simulink model "TosAeb"  (R2021a)
settings: Solver=FixedStepDiscrete  StartTime=0.0  StopTime=inf  FixedStep=0.01  RelTol=1e-3
undefined symbols (not defined in the file or in its folder): ACCModSts, AccSts1, AEB_ObjectMovingOrientation, AEB_ObjectMovingState, CN_BTCActive, CN_CurrentGearR, CN_CurrentGearR_DHT, CN_PTCActive, CN_VDCActive, CN_VSC_EspOff, CN_VSC_FCW_AEB_Error, CN_VSC_FCW_AEB_NoDispay, CN_VSC_FCW_AEB_PerfmDgdtn, CN_VSC_FCW_AEB_TempUnAvlbl, CN_VSC_VehStandstill, CNaccelAvoidMax, CNaccelAvoidMin, CNbrakePedalPress, CNcomfortStrLeftAccelMax, CNcomfortStrLeftAccelMin, CNcomfortStrRightAccelMax, CNcomfortStrRightAccelMin, CNDrvDoorOpen, CNDrvModeEco, CNDrvModeNorm, CNDrvModeOther, CNDrvModeSport, CNDrvSBRreminder, CNNull, CNobjInvalidID, CNPassDoorOpen, CNPassSBRreminder, CNPEBSAccelNegInf, CNPEBSAccelNull, CNPEBSTimeInf, CNPEBSTimeNull, CNSTOPMAX, CNSTOPNULL, CNsubjWidth, CNSwtOFF, CNSwtON, CNTTCMAX, CNTTCNULL, CNVehConfigAEBCycNone, CNVehConfigAEBNone, CNVehConfigAEBPedNone, CNVehConfigFCWNone, CNVehConfigJANone, CollisionSeverity, FALSE, FCWSnvty, JACycObjCalcInfoDefaultParams, JAPedObjCalcInfoDefaultParams, JAVehObjCalcInfoDefaultParams, latCycObjCalcInfoDefaultParams, latPedObjCalcInfoDefaultParams, longObjCalcInfoDefaultParams, ModSts, movingStatus, ObjectType ... (+1112)
libraries: simulink x3031, AEBSFunctionLib x50, Ta_Library x33, AEBSStateMachineLib x23, Mylibrary x12, simulink_extras x7, ObjSelect_lib x3, dspstat3 x2
(part 65 of 102)

== system TosAeb/FcwAebJa_Step_sys/ALG_AEBSystem/ALG_AEBController/AEB_LongTgtInfoCalc/LongObjCalcInfoCalc/AEBS_LongCollisionAssesApp/collisionSeverityHighCalc/collisionSeverityHighCalc1/BrkSysAtribution/FCWSnvtyLate ==
  ObjectMovingState : Inport
  subjVel : Inport  Port=2
  subjLongAccel : Inport  Port=3
  Action Port : ActionPort  ActionPortLabel=case [ HMISettingLate ]:
  Bus Creator : BusCreator  Inputs=3
  Bus Creator1 : BusCreator  Inputs=3
  Bus Creator2 : BusCreator  Inputs=2
  Compare To Constant : simulink/Logic and Bit Operations/Compare To Constant  relop===  const=AEB_ObjectMovingState.Still  OutDataTypeStr=boolean
  Compare To Constant1 : simulink/Logic and Bit Operations/Compare To Constant  relop===  const=AEB_ObjectMovingState.Still  OutDataTypeStr=boolean
  Compare To Constant2 : simulink/Logic and Bit Operations/Compare To Constant  relop===  const=AEB_ObjectMovingState.Still  OutDataTypeStr=boolean
  Constant : Constant  Commented=on  Value=P_AEB_LongCA_SeverityHighDlyTimeConfig
  DelayTimeHigh : Constant  Commented=on  Value=P_AEB_LongCA_SeverityHighBrkDlyTimeHighHmiLate  OutDataTypeStr=single
  DelayTimeLow : Constant  Commented=on  Value=P_AEB_LongCA_SeverityHighBrkDlyTimeLowHmiLate  OutDataTypeStr=single
  SubjAccelThres : simulink/Logic and Bit Operations/Compare To Constant  relop=<  const=P_AEB_LongCA_SeverityHighSubjAccelThes4BrkDlyTimeHmiLate  OutDataTypeStr=boolean
  Switch{,2..4} (4 alike) : Switch  Criteria=u2 ~= 0
  Switch1 : Switch  Commented=on  Criteria=u2 ~= 0
  VelMaxReudceMO : Constant  Value=P_AEB_LongCA_SeverityHighVelMaxReduceMOHmiLate
  VelMaxReudceSO : Constant  Value=P_AEB_LongCA_SeverityHighVelMaxReduceSOHmiLate
  accelGradientMOLookUp : Lookup_n-D  NumberOfTableDimensions=1  BreakpointsForDimension1=P_AEB_LongCA_SeverityHighAccelGredientMOXHmiLate  BeginIndexSearchUsingPreviousIndexResult=on  Table=P_AEB_LongCA_SeverityHighAccelGredientMOYHmiLate  ExtrapMethod=Clip  InternalRulePriority=Speed  RndMeth=Simplest  InputSameDT=on
  accelGradientSOLookUp : Lookup_n-D  NumberOfTableDimensions=1  BreakpointsForDimension1=P_AEB_LongCA_SeverityHighAccelGredientSOXHmiLate  BeginIndexSearchUsingPreviousIndexResult=on  Table=P_AEB_LongCA_SeverityHighAccelGredientSOYHmiLate  ExtrapMethod=Clip  InternalRulePriority=Speed  RndMeth=Simplest  InputSameDT=on
  accelMaxReudceMOLookUp : Lookup_n-D  NumberOfTableDimensions=1  BreakpointsForDimension1=P_AEB_LongCA_SeverityHighAccelMaxDecMOXHmiLate  BeginIndexSearchUsingPreviousIndexResult=on  Table=P_AEB_LongCA_SeverityHighAccelMaxDecMOYHmiLate  ExtrapMethod=Clip  InternalRulePriority=Speed  RndMeth=Simplest  InputSameDT=on
  accelMaxReudceSOLookUp : Lookup_n-D  NumberOfTableDimensions=1  BreakpointsForDimension1=P_AEB_LongCA_SeverityHighAccelMaxDecSOXHmiLate  BeginIndexSearchUsingPreviousIndexResult=on  Table=P_AEB_LongCA_SeverityHighAccelMaxDecSOYHmiLate  ExtrapMethod=Clip  InternalRulePriority=Speed  RndMeth=Simplest  InputSameDT=on
  tBrakeLatencyHighMOLookUp : Lookup_n-D  NumberOfTableDimensions=1  BreakpointsForDimension1=P_AEB_LongCA_SeverityHighDlyTimeMOXHmiLate  BeginIndexSearchUsingPreviousIndexResult=on  Table=P_AEB_LongCA_SeverityHighDlyTimeMOYHmiLate  ExtrapMethod=Clip  InternalRulePriority=Speed  RndMeth=Simplest  InputSameDT=on
  tBrakeLatencyHighSOLookUp : Lookup_n-D  NumberOfTableDimensions=1  BreakpointsForDimension1=P_AEB_LongCA_SeverityHighDlyTimeSOXHmiLate  BeginIndexSearchUsingPreviousIndexResult=on  Table=P_AEB_LongCA_SeverityHighDlyTimeSOYHmiLate  ExtrapMethod=Clip  InternalRulePriority=Speed  RndMeth=Simplest  InputSameDT=on
  tBrakeLatencyLowMOLookUp : Lookup_n-D  NumberOfTableDimensions=1  BreakpointsForDimension1=P_AEB_LongCA_SeverityHighDlyTimeLowMOXHmiLate  BeginIndexSearchUsingPreviousIndexResult=on  Table=P_AEB_LongCA_SeverityHighDlyTimeLowMOYHmiLate  ExtrapMethod=Clip  InternalRulePriority=Speed  RndMeth=Simplest  InputSameDT=on
  tBrakeLatencyLowSOLookUp : Lookup_n-D  NumberOfTableDimensions=1  BreakpointsForDimension1=P_AEB_LongCA_SeverityHighDlyTimeLowSOXHmiLate  BeginIndexSearchUsingPreviousIndexResult=on  Table=P_AEB_LongCA_SeverityHighDlyTimeLowSOYHmiLate  ExtrapMethod=Clip  InternalRulePriority=Speed  RndMeth=Simplest  InputSameDT=on
  brkSys : Outport
  wires:
  Bus Creator2/1 -> brkSys/1
  accelMaxReudceMOLookUp/1 -> Bus Creator1/2  "accelMaxReudce"
  accelGradientMOLookUp/1 -> Bus Creator1/3  "accelGradient"
  VelMaxReudceMO/1 -> Bus Creator1/1  "velMaxReudce"
  Compare To Constant/1 -> Switch/2
  VelMaxReudceSO/1 -> Bus Creator/1  "velMaxReudce"
  Bus Creator/1 -> Switch/1
  Switch/1 -> Bus Creator2/1  "brkSysAtribution"
  Switch3/1 -> Bus Creator2/2  "tBrakeLatency"
  subjLongAccel/1 -> SubjAccelThres/1
  accelGradientSOLookUp/1 -> Bus Creator/3  "accelGradient"
  Bus Creator1/1 -> Switch/3
  ObjectMovingState/1 -> [ObjectMovingState], Compare To Constant/1
  accelMaxReudceSOLookUp/1 -> Bus Creator/2  "accelMaxReudce"
  [ObjectMovingState] -> Compare To Constant1/1
  subjVel/1 -> accelMaxReudceSOLookUp/1, accelGradientSOLookUp/1, accelMaxReudceMOLookUp/1, accelGradientMOLookUp/1, tBrakeLatencyHighSOLookUp/1, tBrakeLatencyHighMOLookUp/1, tBrakeLatencyLowMOLookUp/1, tBrakeLatencyLowSOLookUp/1
  tBrakeLatencyHighMOLookUp/1 -> Switch2/3
  Compare To Constant1/1 -> Switch2/2
  tBrakeLatencyHighSOLookUp/1 -> Switch2/1
  Switch2/1 -> Switch3/3  "tBrakeLatency"
  SubjAccelThres/1 -> Switch3/2
  tBrakeLatencyLowSOLookUp/1 -> Switch4/1
  Compare To Constant2/1 -> Switch4/2
  tBrakeLatencyLowMOLookUp/1 -> Switch4/3
  [ObjectMovingState] -> Compare To Constant2/1
  Switch4/1 -> Switch3/1  "tBrakeLatency"

== system TosAeb/FcwAebJa_Step_sys/ALG_AEBSystem/ALG_AEBController/AEB_LongTgtInfoCalc/LongObjCalcInfoCalc/AEBS_LongCollisionAssesApp/collisionSeverityHighCalc/collisionSeverityHighCalc1/BrkSysAtribution/FCWSnvtyMid ==
  ObjectMovingState : Inport
  subjVel : Inport  Port=2
  subjLongAccel : Inport  Port=3
  Action Port : ActionPort  ActionPortLabel=default:
  Bus Creator : BusCreator  Inputs=3
  Bus Creator1 : BusCreator  Inputs=3
  Bus Creator2 : BusCreator  Inputs=2
  Compare To Constant : simulink/Logic and Bit Operations/Compare To Constant  relop===  const=AEB_ObjectMovingState.Still  OutDataTypeStr=boolean
  Compare To Constant1 : simulink/Logic and Bit Operations/Compare To Constant  relop===  const=AEB_ObjectMovingState.Still  OutDataTypeStr=boolean
  Compare To Constant2 : simulink/Logic and Bit Operations/Compare To Constant  relop===  const=AEB_ObjectMovingState.Still  OutDataTypeStr=boolean
  Constant : Constant  Commented=on  Value=P_AEB_LongCA_SeverityHighDlyTimeConfig
  DelayTimeHigh : Constant  Commented=on  Value=P_AEB_LongCA_SeverityHighBrkDlyTimeHighHmiMid  OutDataTypeStr=single
  DelayTimeLow : Constant  Commented=on  Value=P_AEB_LongCA_SeverityHighBrkDlyTimeLowHmiMid  OutDataTypeStr=single
  SubjAccelThres : simulink/Logic and Bit Operations/Compare To Constant  relop=<  const=P_AEB_LongCA_SeverityHighSubjAccelThes4BrkDlyTimeHmiMid  OutDataTypeStr=boolean
  Switch{,2..4} (4 alike) : Switch  Criteria=u2 ~= 0
  Switch1 : Switch  Commented=on  Criteria=u2 ~= 0
  VelMaxReudceMO : Constant  Value=P_AEB_LongCA_SeverityHighVelMaxReduceMOHmiMid
  VelMaxReudceSO : Constant  Value=P_AEB_LongCA_SeverityHighVelMaxReduceSOHmiMid
  accelGradientMOLookUp : Lookup_n-D  NumberOfTableDimensions=1  BreakpointsForDimension1=P_AEB_LongCA_SeverityHighAccelGredientMOXHmiMid  BeginIndexSearchUsingPreviousIndexResult=on  Table=P_AEB_LongCA_SeverityHighAccelGredientMOYHmiMid  ExtrapMethod=Clip  InternalRulePriority=Speed  RndMeth=Simplest  InputSameDT=on
  accelGradientSOLookUp : Lookup_n-D  NumberOfTableDimensions=1  BreakpointsForDimension1=P_AEB_LongCA_SeverityHighAccelGredientSOXHmiMid  BeginIndexSearchUsingPreviousIndexResult=on  Table=P_AEB_LongCA_SeverityHighAccelGredientSOYHmiMid  ExtrapMethod=Clip  InternalRulePriority=Speed  RndMeth=Simplest  InputSameDT=on
  accelMaxReudceMOLookUp : Lookup_n-D  NumberOfTableDimensions=1  BreakpointsForDimension1=P_AEB_LongCA_SeverityHighAccelMaxDecMOXHmiMid  BeginIndexSearchUsingPreviousIndexResult=on  Table=P_AEB_LongCA_SeverityHighAccelMaxDecMOYHmiMid  ExtrapMethod=Clip  InternalRulePriority=Speed  RndMeth=Simplest  InputSameDT=on
  accelMaxReudceSOLookUp : Lookup_n-D  NumberOfTableDimensions=1  BreakpointsForDimension1=P_AEB_LongCA_SeverityHighAccelMaxDecSOXHmiMid  BeginIndexSearchUsingPreviousIndexResult=on  Table=P_AEB_LongCA_SeverityHighAccelMaxDecSOYHmiMid  ExtrapMethod=Clip  InternalRulePriority=Speed  RndMeth=Simplest  InputSameDT=on
  tBrakeLatencyLowMOLookUp : Lookup_n-D  NumberOfTableDimensions=1  BreakpointsForDimension1=P_AEB_LongCA_SeverityHighDlyTimeLowMOXHmiMid  BeginIndexSearchUsingPreviousIndexResult=on  Table=P_AEB_LongCA_SeverityHighDlyTimeLowMOYHmiMid  ExtrapMethod=Clip  InternalRulePriority=Speed  RndMeth=Simplest  InputSameDT=on
  tBrakeLatencyLowSOLookUp : Lookup_n-D  NumberOfTableDimensions=1  BreakpointsForDimension1=P_AEB_LongCA_SeverityHighDlyTimeLowSOXHmiMid  BeginIndexSearchUsingPreviousIndexResult=on  Table=P_AEB_LongCA_SeverityHighDlyTimeLowSOYHmiMid  ExtrapMethod=Clip  InternalRulePriority=Speed  RndMeth=Simplest  InputSameDT=on
  tBrakeLatencyMOLookUp : Lookup_n-D  NumberOfTableDimensions=1  BreakpointsForDimension1=P_AEB_LongCA_SeverityHighDlyTimeMOXHmiMid  BeginIndexSearchUsingPreviousIndexResult=on  Table=P_AEB_LongCA_SeverityHighDlyTimeMOYHmiMid  ExtrapMethod=Clip  InternalRulePriority=Speed  RndMeth=Simplest  InputSameDT=on
  tBrakeLatencySOLookUp : Lookup_n-D  NumberOfTableDimensions=1  BreakpointsForDimension1=P_AEB_LongCA_SeverityHighDlyTimeSOXHmiMid  BeginIndexSearchUsingPreviousIndexResult=on  Table=P_AEB_LongCA_SeverityHighDlyTimeSOYHmiMid  ExtrapMethod=Clip  InternalRulePriority=Speed  RndMeth=Simplest  InputSameDT=on
  brkSys : Outport
  wires:
  subjVel/1 -> tBrakeLatencyMOLookUp/1, tBrakeLatencySOLookUp/1, tBrakeLatencyLowMOLookUp/1, tBrakeLatencyLowSOLookUp/1, accelGradientMOLookUp/1, accelMaxReudceMOLookUp/1, accelGradientSOLookUp/1, accelMaxReudceSOLookUp/1
  accelMaxReudceSOLookUp/1 -> Bus Creator/2  "accelMaxReudce"
  ObjectMovingState/1 -> [ObjectMovingState], Compare To Constant/1
  Bus Creator1/1 -> Switch/3
  accelGradientSOLookUp/1 -> Bus Creator/3  "accelGradient"
  subjLongAccel/1 -> SubjAccelThres/1
  Switch3/1 -> Bus Creator2/2  "tBrakeLatency"
  Switch/1 -> Bus Creator2/1  "brkSysAtribution"
  Bus Creator/1 -> Switch/1
  VelMaxReudceSO/1 -> Bus Creator/1  "velMaxReudce"
  Compare To Constant/1 -> Switch/2
  VelMaxReudceMO/1 -> Bus Creator1/1  "velMaxReudce"
  accelGradientMOLookUp/1 -> Bus Creator1/3  "accelGradient"
  accelMaxReudceMOLookUp/1 -> Bus Creator1/2  "accelMaxReudce"
  Bus Creator2/1 -> brkSys/1
  Compare To Constant1/1 -> Switch2/2
  tBrakeLatencySOLookUp/1 -> Switch2/1
  tBrakeLatencyMOLookUp/1 -> Switch2/3
  [ObjectMovingState] -> Compare To Constant1/1
  Switch2/1 -> Switch3/3  "tBrakeLatency"
  SubjAccelThres/1 -> Switch3/2
  Switch4/1 -> Switch3/1  "tBrakeLatency"
  [ObjectMovingState] -> Compare To Constant2/1
  tBrakeLatencyLowMOLookUp/1 -> Switch4/3
  tBrakeLatencyLowSOLookUp/1 -> Switch4/1
  Compare To Constant2/1 -> Switch4/2

== system TosAeb/FcwAebJa_Step_sys/ALG_AEBSystem/ALG_AEBController/AEB_LongTgtInfoCalc/LongObjCalcInfoCalc/AEBS_LongCollisionAssesApp/collisionSeverityHighCalc/collisionSeverityHighCalc1/CollisionAssessHighCalcVersion3/CollisionAssessHighCalc1 ==
  accelMaxDec : Inport
  accelGradient : Inport  Port=2
  velMaxReudce : Inport  Port=3
  tBrakeLatency : Inport  Port=4
  vxAbsObj : Inport  Port=5
  axAbsObj : Inport  Port=6
  subjVel : Inport  Port=7
  subjLongAccel : Inport  Port=8
  dxFrontBumper : Inport  Port=9
  dxSecure : Inport  Port=10
  Action Port : ActionPort  ActionPortLabel=else
  Bus Creator : BusCreator  Inputs=8
  Bus Selector : BusSelector  OutputSignals=subjLongAccelFilt,axAbsObj,tBrakeLatency,velMaxReudce,subjVel,vxAbsObj
  Bus Selector2 : BusSelector  OutputSignals=accelMaxDec,subjLongAccelFilt,accelGradient,axAbsObj
  Bus Selector3 : BusSelector  OutputSignals=accelMaxDec,axAbsObj,accelMaxDec
  CalcBrakePhase1 : Subsystem
  CalcBrakePhase2 : Subsystem
  CalcBrakePhase3 : Subsystem
  collisionSeverityHighFlgJudge : Subsystem
  dxWithoutSecureCalc : Subsystem
  subjLongAccelFiltCalc : Subsystem
  collisionSeverityHighFlg : Outport
  wires:
  Bus Selector3/3 -> CalcBrakePhase3/3(accelMaxDec)  "<accelMaxDec>"
  Bus Selector2/1 -> CalcBrakePhase2/1(accelMaxDec)  "<accelMaxDec>"
  tBrakeLatency/1 -> Bus Creator/4  "tBrakeLatency"
  velMaxReudce/1 -> Bus Creator/3  "velMaxReudce"
  accelGradient/1 -> Bus Creator/2  "accelGradient"
  accelMaxDec/1 -> Bus Creator/1  "accelMaxDec"
  subjLongAccelFiltCalc/1(subjLongAccelFilt) -> Bus Creator/8  "subjLongAccelFilt"
  subjVel/1 -> Bus Creator/7  "subjVel"
  axAbsObj/1 -> Bus Creator/6  "axAbsObj"
  vxAbsObj/1 -> Bus Creator/5  "vxAbsObj"
  Bus Selector3/1 -> CalcBrakePhase3/1(a0Ego)  "<accelMaxDec>"
  subjLongAccel/1 -> subjLongAccelFiltCalc/1(subjLongAccel)
  dxWithoutSecureCalc/1(dxWithoutSecure) -> CalcBrakePhase1/7(dx)
  CalcBrakePhase1/4(vtObj) -> CalcBrakePhase2/7(v0Obj)
  CalcBrakePhase1/3(vtEgo) -> CalcBrakePhase2/6(v0Ego)
  [egoCollisionFlgPhase1] -> collisionSeverityHighFlgJudge/1(egoCollisionFlgPhase1)
  [egoCollisionFlgPhase2] -> collisionSeverityHighFlgJudge/2(egoCollisionFlgPhase2)
  CalcBrakePhase3/1(egoCollisionFlg) -> collisionSeverityHighFlgJudge/3(egoCollisionFlgPhase3)
  collisionSeverityHighFlgJudge/1(collisionSeverityHighFlg) -> collisionSeverityHighFlg/1
  CalcBrakePhase1/1(egoCollisionFlg) -> [egoCollisionFlgPhase1]
  CalcBrakePhase2/1(egoCollisionFlg) -> [egoCollisionFlgPhase2]
  CalcBrakePhase2/4(vtObj) -> CalcBrakePhase3/6(v0Obj)
  CalcBrakePhase2/3(vtEgo) -> CalcBrakePhase3/5(v0Ego)
  dxFrontBumper/1 -> dxWithoutSecureCalc/1(dxFrontBumper)
  dxSecure/1 -> dxWithoutSecureCalc/2(dxSecure)
  Bus Creator/1 -> Bus Selector/1, Bus Selector2/1, Bus Selector3/1
  Bus Selector/3 -> CalcBrakePhase1/3(tBrakeLatency)  "<tBrakeLatency>"
  Bus Selector/2 -> CalcBrakePhase1/2(a0Obj)  "<axAbsObj>"
  Bus Selector/4 -> CalcBrakePhase1/4(velMaxReudce)  "<velMaxReudce>"
  Bus Selector/1 -> CalcBrakePhase1/1(a0Ego)  "<subjLongAccelFilt>"
  Bus Selector/5 -> CalcBrakePhase1/5(v0Ego)  "<subjVel>"
  Bus Selector/6 -> CalcBrakePhase1/6(v0Obj)  "<vxAbsObj>"
  Bus Selector2/2 -> CalcBrakePhase2/2(a0Ego)  "<subjLongAccelFilt>"
  Bus Selector2/3 -> CalcBrakePhase2/3(accelGradient)  "<accelGradient>"
  Bus Selector2/4 -> CalcBrakePhase2/4(a0Obj)  "<axAbsObj>"
  Bus Selector3/2 -> CalcBrakePhase3/2(a0Obj)  "<axAbsObj>"
  CalcBrakePhase1/2(restDeltaVel) -> CalcBrakePhase2/5(velMaxReudce)
  CalcBrakePhase2/2(restDeltaVelocity) -> CalcBrakePhase3/4(velMaxReudce)
  CalcBrakePhase1/5(dxt) -> CalcBrakePhase2/8(dx)
  CalcBrakePhase2/5(dxt) -> CalcBrakePhase3/7(dx0)

== system TosAeb/FcwAebJa_Step_sys/ALG_AEBSystem/ALG_AEBController/AEB_LongTgtInfoCalc/LongObjCalcInfoCalc/AEBS_LongCollisionAssesApp/collisionSeverityHighCalc/collisionSeverityHighCalc1/CollisionAssessHighCalcVersion3/CollisionAssessHighCalc2 ==
  accelMaxDec : Inport
  accelGradient : Inport  Port=2
  velMaxReudce : Inport  Port=3
  tBrakeLatency : Inport  Port=4
  vxAbsObj : Inport  Port=5
  axAbsObj : Inport  Port=6
  subjVel : Inport  Port=7
  subjLongAccel : Inport  Port=8
  dxFrontBumper : Inport  Port=9
  dxSecure : Inport  Port=10
  Action Port : ActionPort
  Bus Creator : BusCreator  Inputs=8
  Bus Selector : BusSelector  OutputSignals=subjLongAccelFilt,axAbsObj,tBrakeLatency,velMaxReudce,subjVel,vxAbsObj
  Bus Selector2 : BusSelector  OutputSignals=accelMaxDec,subjLongAccelFilt,accelGradient,axAbsObj
  Bus Selector3 : BusSelector  OutputSignals=accelMaxDec,axAbsObj,accelMaxDec
  CalcBrakePhase1 : Subsystem
  CalcBrakePhase2 : Subsystem
  CalcBrakePhase3 : Subsystem
  Constant : Constant  Value=0
  Constant2 : Constant  Value=0
  collisionSeverityHighFlgJudge : Subsystem
  dxWithoutSecureCalc : Subsystem
  subjLongAccelFiltCalc : Subsystem
  collisionSeverityHighFlg : Outport
  wires:
  Bus Selector3/3 -> CalcBrakePhase3/3(accelMaxDec)  "<accelMaxDec>"
  Bus Selector2/1 -> CalcBrakePhase2/1(accelMaxDec)  "<accelMaxDec>"
  tBrakeLatency/1 -> Bus Creator/4  "tBrakeLatency"
  velMaxReudce/1 -> Bus Creator/3  "velMaxReudce"
  accelGradient/1 -> Bus Creator/2  "accelGradient"
  accelMaxDec/1 -> Bus Creator/1  "accelMaxDec"
  subjLongAccelFiltCalc/1(subjLongAccelFilt) -> Bus Creator/8  "subjLongAccelFilt"
  subjVel/1 -> Bus Creator/7  "subjVel"
  axAbsObj/1 -> Bus Creator/6  "axAbsObj"
  vxAbsObj/1 -> Bus Creator/5  "vxAbsObj"
  Bus Selector3/1 -> CalcBrakePhase3/1(a0Ego)  "<accelMaxDec>"
  subjLongAccel/1 -> subjLongAccelFiltCalc/1(subjLongAccel)
  dxWithoutSecureCalc/1(dxWithoutSecure) -> CalcBrakePhase1/9(dx), CalcBrakePhase3/9(dx), CalcBrakePhase2/10(dx)
  CalcBrakePhase1/6(stObj) -> CalcBrakePhase2/9(s0Obj)
  CalcBrakePhase1/5(vtObj) -> CalcBrakePhase2/8(v0Obj)
  CalcBrakePhase1/3(vtEgo) -> CalcBrakePhase2/6(v0Ego)
  CalcBrakePhase1/4(stEgo) -> CalcBrakePhase2/7(s0Ego)
  [egoCollisionFlgPhase1] -> collisionSeverityHighFlgJudge/1(egoCollisionFlgPhase1)
  [egoCollisionFlgPhase2] -> collisionSeverityHighFlgJudge/2(egoCollisionFlgPhase2)
  CalcBrakePhase3/1(egoCollisionFlg) -> collisionSeverityHighFlgJudge/3(egoCollisionFlgPhase3)
  collisionSeverityHighFlgJudge/1(collisionSeverityHighFlg) -> collisionSeverityHighFlg/1
  CalcBrakePhase1/1(egoCollisionFlg) -> [egoCollisionFlgPhase1]
  CalcBrakePhase2/1(egoCollisionFlg) -> [egoCollisionFlgPhase2]
  CalcBrakePhase2/6(stObj) -> CalcBrakePhase3/8(s0Obj)
  CalcBrakePhase2/5(vtObj) -> CalcBrakePhase3/7(v0Obj)
  CalcBrakePhase2/4(stEgo) -> CalcBrakePhase3/6(s0Ego)
  CalcBrakePhase2/3(vtEgo) -> CalcBrakePhase3/5(v0Ego)
  Constant/1 -> CalcBrakePhase1/8(s0Obj)
  Constant2/1 -> CalcBrakePhase1/6(s0Ego)
  dxFrontBumper/1 -> dxWithoutSecureCalc/1(dxFrontBumper)
  dxSecure/1 -> dxWithoutSecureCalc/2(dxSecure)
  Bus Creator/1 -> Bus Selector/1, Bus Selector2/1, Bus Selector3/1
  Bus Selector/3 -> CalcBrakePhase1/3(tBrakeLatency)  "<tBrakeLatency>"
  Bus Selector/2 -> CalcBrakePhase1/2(a0Obj)  "<axAbsObj>"
  Bus Selector/4 -> CalcBrakePhase1/4(velMaxReudce)  "<velMaxReudce>"
  Bus Selector/1 -> CalcBrakePhase1/1(a0Ego)  "<subjLongAccelFilt>"
  Bus Selector/5 -> CalcBrakePhase1/5(v0Ego)  "<subjVel>"
  Bus Selector/6 -> CalcBrakePhase1/7(v0Obj)  "<vxAbsObj>"
  Bus Selector2/2 -> CalcBrakePhase2/2(a0Ego)  "<subjLongAccelFilt>"
  Bus Selector2/3 -> CalcBrakePhase2/3(accelGradient)  "<accelGradient>"
  Bus Selector2/4 -> CalcBrakePhase2/4(a0Obj)  "<axAbsObj>"
  Bus Selector3/2 -> CalcBrakePhase3/2(a0Obj)  "<axAbsObj>"
  CalcBrakePhase1/2(restDeltaVel) -> CalcBrakePhase2/5(velMaxReudce)
  CalcBrakePhase2/2(restDeltaVelocity) -> CalcBrakePhase3/4(velMaxReudce)

== system TosAeb/FcwAebJa_Step_sys/ALG_AEBSystem/ALG_AEBController/AEB_LongTgtInfoCalc/LongObjCalcInfoCalc/AEBS_LongCollisionAssesApp/collisionSeverityHighCalc/collisionSeverityHighCalc1/CollisionAssessHighCalcVersion3/CollisionAssessHighCalcInvalid ==
  accelGradient : Inport
  accelMaxDec : Inport  Port=2
  Compare To Constant : simulink/Logic and Bit Operations/Compare To Constant  relop=>=  const=CNNull  OutDataTypeStr=boolean
  Compare To Constant1 : simulink/Logic and Bit Operations/Compare To Constant  relop=>=  const=CNNull  OutDataTypeStr=boolean
  Logical Operator : Logic  Operator=OR  AllPortsSameDT=off  OutDataTypeStr=boolean
  Invalid : Outport
  wires:
  Logical Operator/1 -> Invalid/1
  accelGradient/1 -> Compare To Constant/1
  accelMaxDec/1 -> Compare To Constant1/1
  Compare To Constant/1 -> Logical Operator/1
  Compare To Constant1/1 -> Logical Operator/2

== system TosAeb/FcwAebJa_Step_sys/ALG_AEBSystem/ALG_AEBController/AEB_LongTgtInfoCalc/LongObjCalcInfoCalc/AEBS_LongCollisionAssesApp/collisionSeverityHighCalc/collisionSeverityHighCalc1/CollisionAssessHighCalcVersion3/If Action Subsystem ==
  Action Port : ActionPort  ActionPortLabel=if(u1)
  Constant : Constant  Value=false
  collisionSeverityHighFlg : Outport
  wires:
  Constant/1 -> collisionSeverityHighFlg/1

== system TosAeb/FcwAebJa_Step_sys/ALG_AEBSystem/ALG_AEBController/AEB_LongTgtInfoCalc/LongObjCalcInfoCalc/AEBS_LongCollisionAssesApp/collisionSeverityHighCalc/collisionSeverityHighCalc2/collisionSeverityHighPhase1/CollisionSeverityHighPhase1Judge ==
  note: if distEgophase1 > (distObjphase1 + dxRelTargetObjCalc - dxSecure)
      | 	collisionDangerLevel3Fullfilled = true;
      | else
      | 	collisionDangerLevel3Fullfilled = false;
  distEgophase1 : Inport
  distObjphase1 : Inport  Port=2
  dxFrontBumper : Inport  Port=3
  dxSecure : Inport  Port=4
  Add : Sum  Inputs=++-
  Relational Operator : RelationalOperator  Operator=>  OutDataTypeStr=boolean  RndMeth=Simplest
  collisionSeverityHighFlgPhase1 : Outport
  wires:
  dxFrontBumper/1 -> Add/2  "dxRelTargetObjCalc"
  distObjphase1/1 -> Add/1  "distObjphase1"
  dxSecure/1 -> Add/3  "dxSecure"
  Add/1 -> Relational Operator/2
  distEgophase1/1 -> Relational Operator/1  "distEgophase1"
  Relational Operator/1 -> collisionSeverityHighFlgPhase1/1  "collisionSeverityHighFlgPhase1"

== system TosAeb/FcwAebJa_Step_sys/ALG_AEBSystem/ALG_AEBController/AEB_LongTgtInfoCalc/LongObjCalcInfoCalc/AEBS_LongCollisionAssesApp/collisionSeverityHighCalc/collisionSeverityHighCalc2/collisionSeverityHighPhase1/Phase1DistCalc ==
  tWorkPhase1 : Inport
  tObjStopPhase1 : Inport  Port=2
  subjVel : Inport  Port=3
  subjLongAccel : Inport  Port=4
  vxAbsObj : Inport  Port=5
  axAbsObj : Inport  Port=6
  Add : Sum
  Add1 : Sum
  DocBlock : simulink/Model-Wide Utilities/DocBlock  DocumentType=Text
      content=|
      | Type your documentation here
  Gain : Gain  Gain=0.5
  Gain1 : Gain  Gain=0.5
  Math Function2 : Math  Operator=square  SignedPower=on  SaturateOnIntegerOverflow=on
  Math Function3 : Math  Operator=square  SignedPower=on  SaturateOnIntegerOverflow=on
  MinMax : MinMax  Inputs=2
  Product{,1..3} (4 alike) : Product
  distEgoPhase1 : Outport
  distTargetPhase1 : Outport  Port=2
  wires:
  subjLongAccel/1 -> Product/2  "subjAccel"
  tWorkPhase1/1 -> Math Function2/1, Product1/2, MinMax/1  "tWorkPhase1"
  Product/1 -> Gain/1
  subjVel/1 -> Product1/1  "subjVel"
  Gain/1 -> Add/2
  Product1/1 -> Add/1
  tObjStopPhase1/1 -> MinMax/2  "tObjStop"
  Add/1 -> distEgoPhase1/1  "distEgophase1"
  Product3/1 -> Add1/1
  Gain1/1 -> Add1/2
  vxAbsObj/1 -> Product3/1  "vxAbsTargetObjCalc"
  Product2/1 -> Gain1/1
  MinMax/1 -> Math Function3/1, Product3/2  "tWorkPhase1Target"
  axAbsObj/1 -> Product2/2  "axAbsTargetObjCalc"
  Add1/1 -> distTargetPhase1/1  "distTargetphase1"
  Math Function2/1 -> Product/1
  Math Function3/1 -> Product2/1

== system TosAeb/FcwAebJa_Step_sys/ALG_AEBSystem/ALG_AEBController/AEB_LongTgtInfoCalc/LongObjCalcInfoCalc/AEBS_LongCollisionAssesApp/collisionSeverityHighCalc/collisionSeverityHighCalc2/collisionSeverityHighPhase1/Phase1TimeCalc ==
  velMaxReudce : Inport
  subjLongAccel : Inport  Port=2
  subjVel : Inport  Port=3
  vxAbsObj : Inport  Port=4
  axAbsObj : Inport  Port=5
  BrakeLatencyTimeCalc : Subsystem
  Bus Creator : BusCreator  Inputs=5
  Bus Selector1 : BusSelector  OutputSignals=subjAccel,velMaxReudce,subjVel
  Bus Selector3 : BusSelector  OutputSignals=subjAccel,subjVel
  Bus Selector4 : BusSelector  OutputSignals=subjAccel
  Bus Selector6 : BusSelector  OutputSignals=axAbsTargetObjCalc,vxAbsTargetObjCalc
  TimeWorkPhase1Calc : Subsystem
  tEgoStopWorkPhase1 : Subsystem
  tMaxReducePhase1Calc : Subsystem
  tObjStopCalc : Subsystem  TreatAsAtomicUnit=on
  tWorkPhase1 : Outport
  tObjStopPhase1 : Outport  Port=2
  wires:
  tEgoStopWorkPhase1/1(tEgoStop) -> TimeWorkPhase1Calc/2(tEgoStop)  "tEgoStop"
  Bus Selector3/2 -> tEgoStopWorkPhase1/2(subjVel)  "<subjVel>"
  Bus Selector3/1 -> tEgoStopWorkPhase1/1(subjLongAccel)  "<subjAccel>"
  tMaxReducePhase1Calc/1(tMaxReduce) -> TimeWorkPhase1Calc/1(tMaxReduce)  "tMaxReduce"
  Bus Selector1/3 -> tMaxReducePhase1Calc/3(subjVel)  "<subjVel>"
  Bus Selector1/2 -> tMaxReducePhase1Calc/2(velMaxReudce)  "<velMaxReudce>"
  Bus Selector1/1 -> tMaxReducePhase1Calc/1(subjLongAccel)  "<subjAccel>"
  tObjStopCalc/1(tStop) -> tObjStopPhase1/1
  subjVel/1 -> Bus Creator/3  "subjVel"
  subjLongAccel/1 -> Bus Creator/2  "subjAccel"
  velMaxReudce/1 -> Bus Creator/1  "velMaxReudce"
  vxAbsObj/1 -> Bus Creator/4  "vxAbsTargetObjCalc"
  axAbsObj/1 -> Bus Creator/5  "axAbsTargetObjCalc"
  Bus Creator/1 -> Bus Selector1/1, Bus Selector3/1, Bus Selector4/1, Bus Selector6/1
  Bus Selector6/2 -> tObjStopCalc/2(velAbs)  "<vxAbsTargetObjCalc>"
  Bus Selector6/1 -> tObjStopCalc/1(accelAbs)  "<axAbsTargetObjCalc>"
  Bus Selector4/1 -> BrakeLatencyTimeCalc/1(subjLongAccel)  "<subjAccel>"
  BrakeLatencyTimeCalc/1(tBrakeLatency) -> TimeWorkPhase1Calc/3(tBrakeLatency)  "tBrakeLatency"
  TimeWorkPhase1Calc/1(tWorkPhase1) -> tWorkPhase1/1

== system TosAeb/FcwAebJa_Step_sys/ALG_AEBSystem/ALG_AEBController/AEB_LongTgtInfoCalc/LongObjCalcInfoCalc/AEBS_LongCollisionAssesApp/collisionSeverityHighCalc/collisionSeverityHighCalc2/collisionSeverityHighPhase2/DistPhase2Calc ==
  accelGradient : Inport
  subVelWorkPhase1 : Inport  Port=2
  subjLongAccelWorkPhase1 : Inport  Port=3
  vxAbsWorkPhase1Target : Inport  Port=4
  axAbsWorkPhase1Target : Inport  Port=5
  tWorkPhase2 : Inport  Port=6
  tObjStopWorkPhase2 : Inport  Port=7
  Add : Sum  Inputs=+++
  Add1 : Sum
  Gain : Gain  Gain=0.5
  Gain1 : Gain  Gain=0.5
  Gain2 : Gain  Gain=1/6
  Math Function2 : Math  Operator=square  SignedPower=on  SaturateOnIntegerOverflow=on
  Math Function3 : Math  Operator=square  SignedPower=on  SaturateOnIntegerOverflow=on
  MinMax : MinMax  Inputs=2
  Product{,1..3} (4 alike) : Product
  Product4 : Product  Inputs=4
  distEgophase2 : Outport
  distTargetphase2 : Outport  Port=2
  wires:
  subjLongAccelWorkPhase1/1 -> Product/2
  tWorkPhase2/1 -> Product4/2, Math Function2/1, MinMax/1, Product1/2, Product4/4, Product4/3
  Product/1 -> Gain/1
  Gain/1 -> Add/3
  Product1/1 -> Add/2
  tObjStopWorkPhase2/1 -> MinMax/2
  Add/1 -> distEgophase2/1
  Product3/1 -> Add1/1
  Gain1/1 -> Add1/2
  vxAbsWorkPhase1Target/1 -> Product3/1
  Product2/1 -> Gain1/1
  MinMax/1 -> Math Function3/1, Product3/2
  axAbsWorkPhase1Target/1 -> Product2/2
  Add1/1 -> distTargetphase2/1
  Math Function2/1 -> Product/1
  Math Function3/1 -> Product2/1
  subVelWorkPhase1/1 -> Product1/1
  Product4/1 -> Gain2/1
  Gain2/1 -> Add/1
  accelGradient/1 -> Product4/1

== system TosAeb/FcwAebJa_Step_sys/ALG_AEBSystem/ALG_AEBController/AEB_LongTgtInfoCalc/LongObjCalcInfoCalc/AEBS_LongCollisionAssesApp/collisionSeverityHighCalc/collisionSeverityHighCalc2/collisionSeverityHighPhase2/EgoObjAfterPhase1 ==
  tWorkphase1 : Inport
  distTargetphase1 : Inport  Port=2
  distEgophase1 : Inport  Port=3
  subjVel : Inport  Port=4
  subjLongAccel : Inport  Port=5
  vxAbsObj : Inport  Port=6
  axAbsObj : Inport  Port=7
  dxFrontBumper : Inport  Port=8
  Add : Sum
  Add1 : Sum
  Add2 : Sum  Inputs=++-
  Product : Product
  Product1 : Product
  subjVelWorkPhase1 : Outport
  subjLongAccelWorkPhase1 : Outport  Port=2
  vxAbsWorkPhase1Target : Outport  Port=3
  axAbsWorkPhase1Target : Outport  Port=4
  distRelWorkPhase1 : Outport  Port=5
  wires:
  Product/1 -> Add/2
  subjLongAccel/1 -> subjLongAccelWorkPhase1/1, Product/2
  tWorkphase1/1 -> Product1/1, Product/1
  distEgophase1/1 -> Add2/3
  Add2/1 -> distRelWorkPhase1/1
  dxFrontBumper/1 -> Add2/2
  distTargetphase1/1 -> Add2/1
  subjVel/1 -> Add/1
  Add/1 -> subjVelWorkPhase1/1
  vxAbsObj/1 -> Add1/2
  Add1/1 -> vxAbsWorkPhase1Target/1
  Product1/1 -> Add1/1
  axAbsObj/1 -> axAbsWorkPhase1Target/1, Product1/2

== system TosAeb/FcwAebJa_Step_sys/ALG_AEBSystem/ALG_AEBController/AEB_LongTgtInfoCalc/LongObjCalcInfoCalc/AEBS_LongCollisionAssesApp/collisionSeverityHighCalc/collisionSeverityHighCalc2/collisionSeverityHighPhase2/Phase2TimeCalc ==
  subjLongAccelWorkPhase1 : Inport
  subjVelWorkPhase1 : Inport  Port=2
  vxAbsWorkPhase1Target : Inport  Port=3
  axAbsWorkPhase1Target : Inport  Port=4
  velMaxReudce : Inport  Port=5
  accelGradient : Inport  Port=6
  accelMaxReudce : Inport  Port=7
  Bus Creator : BusCreator  Inputs=7
  Bus Selector1 : BusSelector  OutputSignals=subjAccelWorkPhase1,velMaxReudce,subjVelWorkPhase1
  Bus Selector2 : BusSelector  OutputSignals=axAbsWorkPhase1Target,vxAbsWorkPhase1Target
  Bus Selector5 : BusSelector  OutputSignals=subjVelWorkPhase1,subjAccelWorkPhase1
  Bus Selector6 : BusSelector  OutputSignals=subjAccelWorkPhase1,accelMaxReudce,accelGradient
  TimeEgoStopCalc : Subsystem
  TimeGradientPhase2Calc : Subsystem
  TimeWorkPhase2Calc : Subsystem
  tMaxReducePhase2Calc : Subsystem
  tObjStopCalc : Subsystem  TreatAsAtomicUnit=on
  tWorkPhase2 : Outport
  tObjStopWorkPhase2 : Outport  Port=2
  wires:
  TimeWorkPhase2Calc/1(tWorkPhase2) -> tWorkPhase2/1
  velMaxReudce/1 -> Bus Creator/5  "velMaxReudce"
  vxAbsWorkPhase1Target/1 -> Bus Creator/3  "vxAbsWorkPhase1Target"
  accelGradient/1 -> Bus Creator/6  "accelGradient"
  subjVelWorkPhase1/1 -> Bus Creator/2  "subjVelWorkPhase1"
  subjLongAccelWorkPhase1/1 -> Bus Creator/1  "subjAccelWorkPhase1"
  Bus Creator/1 -> Bus Selector2/1, Bus Selector6/1, Bus Selector5/1, Bus Selector1/1
  axAbsWorkPhase1Target/1 -> Bus Creator/4  "axAbsWorkPhase1Target"
  accelMaxReudce/1 -> Bus Creator/7  "accelMaxReudce"
  Bus Selector1/1 -> tMaxReducePhase2Calc/1(subjLongAccelWorkPhase1)  "<subjAccelWorkPhase1>"
  Bus Selector1/2 -> tMaxReducePhase2Calc/2(velMaxReudce)  "<velMaxReudce>"
  Bus Selector1/3 -> tMaxReducePhase2Calc/3(subjVelWorkPhase1)  "<subjVelWorkPhase1>"
  tMaxReducePhase2Calc/1(tMaxReduce) -> TimeWorkPhase2Calc/1(tMaxReduce)  "tMaxReduce"
  TimeEgoStopCalc/1(tEgoStop) -> TimeWorkPhase2Calc/2(tEgoStop)  "tEgoStop"
  Bus Selector5/2 -> TimeEgoStopCalc/2(subjLongAccelWorkPhase1)  "<subjAccelWorkPhase1>"
  Bus Selector5/1 -> TimeEgoStopCalc/1(subjVelWorkPhase1)  "<subjVelWorkPhase1>"
  TimeGradientPhase2Calc/1(tGradientPhase2) -> TimeWorkPhase2Calc/3(tGradientPhase2)  "tGradientPhase2"
  Bus Selector6/3 -> TimeGradientPhase2Calc/3(accelGradient)  "<accelGradient>"
  Bus Selector6/2 -> TimeGradientPhase2Calc/2(accelMaxReudce)  "<accelMaxReudce>"
  Bus Selector6/1 -> TimeGradientPhase2Calc/1(subjLongAccelWorkPhase1)  "<subjAccelWorkPhase1>"
  Bus Selector2/1 -> tObjStopCalc/1(accelAbs)  "<axAbsWorkPhase1Target>"
  Bus Selector2/2 -> tObjStopCalc/2(velAbs)  "<vxAbsWorkPhase1Target>"
  tObjStopCalc/1(tStop) -> tObjStopWorkPhase2/1

== system TosAeb/FcwAebJa_Step_sys/ALG_AEBSystem/ALG_AEBController/AEB_LongTgtInfoCalc/LongObjCalcInfoCalc/AEBS_LongCollisionAssesApp/collisionSeverityHighCalc/collisionSeverityHighCalc2/collisionSeverityHighPhase2/collisionSeverityHighPhase2FlgCalc ==
  note: if distEgophase2 > (distTargetPhase2 + distRelWorkPhase1 - dxSecure)
      | 	collisionDgrLvl3Phase2 = true;
      | else
      | 	collisionDgrLvl3Phase2 = false;
  distEgophase2 : Inport
  distTargetPhase2 : Inport  Port=2
  distRelWorkPhase1 : Inport  Port=3
  dxSecure : Inport  Port=4
  Add : Sum  Inputs=++-
  Relational Operator : RelationalOperator  Operator=>  OutDataTypeStr=boolean  RndMeth=Simplest
  collisionSeverityHighPhase2 : Outport
  wires:
  distEgophase2/1 -> Relational Operator/1
  Relational Operator/1 -> collisionSeverityHighPhase2/1  "collisionDgrLvl3Phase2"
  dxSecure/1 -> Add/3
  distTargetPhase2/1 -> Add/1
  Add/1 -> Relational Operator/2
  distRelWorkPhase1/1 -> Add/2

== system TosAeb/FcwAebJa_Step_sys/ALG_AEBSystem/ALG_AEBController/AEB_LongTgtInfoCalc/LongObjCalcInfoCalc/AEBS_LongCollisionAssesApp/collisionSeverityHighCalc/collisionSeverityHighCalc2/collisionSeverityHighPhase3/CollisionSeverityHighPhase3Calc ==
  note: if distEgoPhase3 > (distTargetPhase3 + distRelWorkPhase2 - dxSecure)
      | 	collisionDangerLevel3Phase3 = true;
      | else
      | 	collisionDangerLevel3Phase3 = false;
  distEgoPhase3 : Inport
  distTargetPhase3 : Inport  Port=2
  distRelWorkPhase2 : Inport  Port=3
  dxSecure : Inport  Port=4
  Add : Sum  Inputs=++-
  Relational Operator : RelationalOperator  Operator=>  OutDataTypeStr=boolean  RndMeth=Simplest
  collisionSeverityHighPhase3 : Outport
  wires:
  distRelWorkPhase2/1 -> Add/2
  dxSecure/1 -> Add/3
  distTargetPhase3/1 -> Add/1
  Add/1 -> Relational Operator/2
  distEgoPhase3/1 -> Relational Operator/1
  Relational Operator/1 -> collisionSeverityHighPhase3/1  "collisionSeverityHighPhase3"

== system TosAeb/FcwAebJa_Step_sys/ALG_AEBSystem/ALG_AEBController/AEB_LongTgtInfoCalc/LongObjCalcInfoCalc/AEBS_LongCollisionAssesApp/collisionSeverityHighCalc/collisionSeverityHighCalc2/collisionSeverityHighPhase3/DistPhase3Calc ==
  subjLongAccelWorkPhase2 : Inport
  subVelWorkPhase2 : Inport  Port=2
  vxAbsWorkPhase2Target : Inport  Port=3
  axAbsWorkPhase2Target : Inport  Port=4
  tWorkPhase3 : Inport  Port=5
  tObjStopWorkPhase3 : Inport  Port=6
  Add : Sum
  Add1 : Sum
  Display{1..5} (5 alike) : Display
  Gain : Gain  Gain=0.5
  Gain1 : Gain  Gain=0.5
  Math Function2 : Math  Operator=square  SignedPower=on  SaturateOnIntegerOverflow=on
  Math Function3 : Math  Operator=square  SignedPower=on  SaturateOnIntegerOverflow=on
  MinMax : MinMax  Inputs=2
  Product{,1..3} (4 alike) : Product
  distEgophase3 : Outport
  distTargetPhase3 : Outport  Port=2
  wires:
  subjLongAccelWorkPhase2/1 -> Product/2
  tWorkPhase3/1 -> Math Function2/1, MinMax/1, Display3/1, Product1/2
  Product/1 -> Gain/1
  vxAbsWorkPhase2Target/1 -> Product3/1
  Gain/1 -> Add/2, Display4/1
  Gain1/1 -> Add1/2
  tObjStopWorkPhase3/1 -> MinMax/2
  axAbsWorkPhase2Target/1 -> Product2/2
  MinMax/1 -> Math Function3/1, Product3/2
  subVelWorkPhase2/1 -> Product1/1, Display1/1
  Add/1 -> distEgophase3/1, Display2/1  "distEgophase3"
  Add1/1 -> distTargetPhase3/1  "distTargetPhase3"
  Product3/1 -> Add1/1
  Product2/1 -> Gain1/1
  Product1/1 -> Add/1, Display5/1
  Math Function2/1 -> Product/1
  Math Function3/1 -> Product2/1

== system TosAeb/FcwAebJa_Step_sys/ALG_AEBSystem/ALG_AEBController/AEB_LongTgtInfoCalc/LongObjCalcInfoCalc/AEBS_LongCollisionAssesApp/collisionSeverityHighCalc/collisionSeverityHighCalc2/collisionSeverityHighPhase3/EgoObjAfterPhase2 ==
  subjVelWorkPhase1 : Inport
  tWorkPhase2 : Inport  Port=2
  axAbsWorkPhase1Target : Inport  Port=3
  subjLongAccelWorkPhase1 : Inport  Port=4
  vxAbsWorkPhase1Target : Inport  Port=5
  distTargetPhase2 : Inport  Port=6
  dxRelWorkPhase1 : Inport  Port=7
  distEgoPhase2 : Inport  Port=8
  accelGradient : Inport  Port=9
  Add : Sum  Inputs=+++
  Add1 : Sum
  Add3 : Sum  Inputs=++-
  Bus Creator : BusCreator  Inputs=6
  Bus Selector : BusSelector  OutputSignals=subjVelWorkPhase1,subjAccelWorkPhase1,tWorkPhase2,accelGradient,subjAccelWorkPhase1
  Bus Selector1 : BusSelector  OutputSignals=vxAbsWorkPhase1Target,axAbsWorkPhase1Target,tWorkPhase2,axAbsWorkPhase1Target
  Gain : Gain  Gain=0.5
  Math Function : Math  Operator=square  SignedPower=on  SaturateOnIntegerOverflow=on
  Product : Product
  Product1 : Product
  Product3 : Product
  subjVelWorkPhase2 : Outport
  subjLongAccelWorkPhase2 : Outport  Port=2
  vxAbsWorkPhase2Target : Outport  Port=3
  axAbsWorkPhase2Target : Outport  Port=4
  distRelWorkPhase2 : Outport  Port=5
  wires:
  Product/1 -> Add/2
  Add/1 -> subjVelWorkPhase2/1
  Add1/1 -> vxAbsWorkPhase2Target/1
  Add3/1 -> distRelWorkPhase2/1
  Math Function/1 -> Product3/1
  Product3/1 -> Gain/1
  distEgoPhase2/1 -> Add3/3
  dxRelWorkPhase1/1 -> Add3/2
  distTargetPhase2/1 -> Add3/1
  subjVelWorkPhase1/1 -> Bus Creator/1  "subjVelWorkPhase1"
  tWorkPhase2/1 -> Bus Creator/2  "tWorkPhase2"
  axAbsWorkPhase1Target/1 -> Bus Creator/3  "axAbsWorkPhase1Target"
  subjLongAccelWorkPhase1/1 -> Bus Creator/4  "subjAccelWorkPhase1"
  vxAbsWorkPhase1Target/1 -> Bus Creator/5  "vxAbsWorkPhase1Target"
  accelGradient/1 -> Bus Creator/6  "accelGradient"
  Bus Creator/1 -> Bus Selector1/1, Bus Selector/1
  Bus Selector/1 -> Add/1  "<subjVelWorkPhase1>"
  Bus Selector/2 -> Product/1  "<subjAccelWorkPhase1>"
  Bus Selector/3 -> Math Function/1, Product/2  "<tWorkPhase2>"
  Bus Selector/4 -> Product3/2  "<accelGradient>"
  Gain/1 -> Add/3
  Bus Selector1/3 -> Product1/2  "<tWorkPhase2>"
  Bus Selector1/2 -> Product1/1  "<axAbsWorkPhase1Target>"
  Product1/1 -> Add1/2
  Bus Selector1/1 -> Add1/1  "<vxAbsWorkPhase1Target>"
  Bus Selector1/4 -> axAbsWorkPhase2Target/1  "<axAbsWorkPhase1Target>"
  Bus Selector/5 -> subjLongAccelWorkPhase2/1  "<subjAccelWorkPhase1>"

== system TosAeb/FcwAebJa_Step_sys/ALG_AEBSystem/ALG_AEBController/AEB_LongTgtInfoCalc/LongObjCalcInfoCalc/AEBS_LongCollisionAssesApp/collisionSeverityHighCalc/collisionSeverityHighCalc2/collisionSeverityHighPhase3/Phase3TimeCalc ==
  subjVelWorkPhase2 : Inport
  subjLongAccelWorkPhase2 : Inport  Port=2
  vxAbsWorkPhase2Target : Inport  Port=3
  axAbsWorkPhase2Target : Inport  Port=4
  accelMaxReduce : Inport  Port=5
  velMaxReudce : Inport  Port=6
  Bus Creator : BusCreator  Inputs=6
  Bus Selector : BusSelector  OutputSignals=subjAccelWorkPhase2,velMaxReudce,subjVelWorkPhase2
  Bus Selector1 : BusSelector  OutputSignals=accelMaxReduce,subjVelWorkPhase2
  Bus Selector2 : BusSelector  OutputSignals=subjVelWorkPhase2,vxAbsWorkPhase2Target,accelMaxReduce,axAbsWorkPhase2Target
  Bus Selector5 : BusSelector  OutputSignals=axAbsWorkPhase2Target,vxAbsWorkPhase2Target
  TimeEgoStopCalc : Subsystem
  TimeWorkPhase3Calc : Subsystem
  tBrakeConstCalc : Subsystem
  tMaxReduceCalc : Subsystem
  tObjStopCalc : Subsystem  TreatAsAtomicUnit=on
  tWorkPhase3 : Outport
  tObjStopWorkPhase3 : Outport  Port=2
  wires:
  axAbsWorkPhase2Target/1 -> Bus Creator/4  "axAbsWorkPhase2Target"
  accelMaxReduce/1 -> Bus Creator/5  "accelMaxReduce"
  vxAbsWorkPhase2Target/1 -> Bus Creator/3  "vxAbsWorkPhase2Target"
  subjLongAccelWorkPhase2/1 -> Bus Creator/2  "subjAccelWorkPhase2"
  velMaxReudce/1 -> Bus Creator/6  "velMaxReudce"
  subjVelWorkPhase2/1 -> Bus Creator/1  "subjVelWorkPhase2"
  Bus Creator/1 -> Bus Selector2/1, Bus Selector5/1, Bus Selector1/1, Bus Selector/1
  TimeWorkPhase3Calc/1(tWorkPhase3) -> tWorkPhase3/1  "tWorkPhase3"
  Bus Selector/1 -> tMaxReduceCalc/1(subjLongAccelWorkPhase2)  "<subjAccelWorkPhase2>"
  Bus Selector/2 -> tMaxReduceCalc/2(velMaxReudce)  "<velMaxReudce>"
  Bus Selector/3 -> tMaxReduceCalc/3(subjVelWorkPhase2)  "<subjVelWorkPhase2>"
  tMaxReduceCalc/1(tMaxReduce) -> TimeWorkPhase3Calc/1(tMaxReduce)  "tMaxReduce"
  Bus Selector1/1 -> TimeEgoStopCalc/1(accelMaxReduce)  "<accelMaxReduce>"
  Bus Selector1/2 -> TimeEgoStopCalc/2(subjVelWorkPhase2)  "<subjVelWorkPhase2>"
  TimeEgoStopCalc/1(tEgoStop) -> TimeWorkPhase3Calc/2(tEgoStop)  "tEgoStop"
  tBrakeConstCalc/1(tBrakeConst) -> TimeWorkPhase3Calc/3(tBrakeConst)  "tBrakeConst"
  Bus Selector2/4 -> tBrakeConstCalc/4(axAbsWorkPhase2Target)  "<axAbsWorkPhase2Target>"
  Bus Selector2/3 -> tBrakeConstCalc/3(accelMaxReduce)  "<accelMaxReduce>"
  Bus Selector2/2 -> tBrakeConstCalc/2(vxAbsWorkPhase2Target)  "<vxAbsWorkPhase2Target>"
  Bus Selector2/1 -> tBrakeConstCalc/1(subjVelWorkPhase2)  "<subjVelWorkPhase2>"
  tObjStopCalc/1(tStop) -> tObjStopWorkPhase3/1  "tObjStopWorkPhase3"
  Bus Selector5/2 -> tObjStopCalc/2(velAbs)  "<vxAbsWorkPhase2Target>"
  Bus Selector5/1 -> tObjStopCalc/1(accelAbs)  "<axAbsWorkPhase2Target>"

== system TosAeb/AEB_Objsel/TA_Processor/AEB_ObjSelect/TargetInfoCheck/Data_precess/TA_EffectCalculate/Obj_FilterFlgCalculate/JA_ObjFilter/CollisionProbCalc/Subsystem/ObjBoundingBox ==
  ObjLengthCorr : Inport  OutDataTypeStr=single
  ObjWidthCorr : Inport  Port=2  OutDataTypeStr=single
  dxPreRefPointObj : Inport  Port=3  OutDataTypeStr=single
  dyPreRefPointObj : Inport  Port=4  OutDataTypeStr=single
  sincosCoef : Inport  Port=5
  Bus Creator : BusCreator  Inputs=5
  Mux : Mux
  Mux1 : Mux
  ObjLLCoordinate : Subsystem
  ObjLUCoordinate : Subsystem
  ObjRLCoordinate : Subsystem
  ObjRUCoordinate : Subsystem
  objDxPre : Outport
  objDyPre : Outport  Port=2
  wires:
  ObjLengthCorr/1 -> Bus Creator/1  "ObjLengthCorr"
  ObjWidthCorr/1 -> Bus Creator/2  "ObjWidthCorr"
  dxPreRefPointObj/1 -> Bus Creator/3  "dxPreRefPointObj"
  dyPreRefPointObj/1 -> Bus Creator/4  "dyPreRefPointObj"
  sincosCoef/1 -> Bus Creator/5  "sincosCoef"
  Bus Creator/1 -> ObjRLCoordinate/1(inputSignals), ObjRUCoordinate/1(inputSignals), ObjLLCoordinate/1(inputSignals), ObjLUCoordinate/1(inputSignals)
  ObjLUCoordinate/1(dxPreLUObj) -> [dxPreLUObj]  "dxPreLUObj"
  ObjLUCoordinate/2(dyPreLUobj) -> [dyPreLUObj]  "dyPreLUObj"
  ObjLLCoordinate/1(dxPre_LLObj) -> [dxPreLLObj]  "dxPreLLObj"
  ObjLLCoordinate/2(dyPreLLObj) -> [dyPreLLObj]  "dyPreLLObj"
  ObjRUCoordinate/1(dxPreRUObj) -> [dxPreRUObj]  "dxPreRUObj"
  ObjRUCoordinate/2(dyPreRUObj) -> [dyPreRUObj]  "dyPreRUObj"
  ObjRLCoordinate/1(dxPreRLObj) -> [dxPreRLObj]  "dxPreRLObj"
  ObjRLCoordinate/2(dyPreRLObj) -> [dyPreRLObj]  "dyPreRLObj"
  Mux/1 -> objDxPre/1
  Mux1/1 -> objDyPre/1
  [dxPreRUObj] -> Mux/1  "dxPreRUObj"
  [dxPreLUObj] -> Mux/2
  [dxPreLLObj] -> Mux/3
  [dxPreRLObj] -> Mux/4
  [dyPreRUObj] -> Mux1/1
  [dyPreLUObj] -> Mux1/2
  [dyPreLLObj] -> Mux1/3
  [dyPreRLObj] -> Mux1/4

== system TosAeb/AEB_Objsel/TA_Processor/AEB_ObjSelect/TargetInfoCheck/Data_precess/TA_EffectCalculate/Obj_FilterFlgCalculate/JA_ObjFilter/CollisionProbCalc/Subsystem/ObjBoundingBoxRebuild ==
  subjDxPre : Inport
  subjDyPre : Inport  Port=2
  objDxPre : Inport  Port=3
  objDyPre : Inport  Port=4
  Demux{,1..3} (4 alike) : Demux
  Mux{,1..3} (4 alike) : Mux
  Subsystem : Subsystem
  Subsystem1 : Subsystem
  Subsystem2 : Subsystem
  Subsystem3 : Subsystem
  Out1 : Outport
  Out2 : Outport  Port=2
  Out3 : Outport  Port=3
  Out4 : Outport  Port=4
  wires:
  subjDxPre/1 -> Demux/1
  subjDyPre/1 -> Demux1/1
  objDxPre/1 -> Demux2/1
  objDyPre/1 -> Demux3/1
  Demux/1 -> [SubDxFR]
  Demux/2 -> [SubDxFL]
  Demux/3 -> [SubDxRL]
  Demux/4 -> [SubDxRR]
  Demux1/1 -> [SubDyFR]
  Demux1/2 -> [SubDyFL]
  Demux1/3 -> [SubDyRL]
  Demux1/4 -> [SubDyRR]
  Demux2/1 -> [ObjDxFR]
  Demux2/2 -> [ObjDxFL]
  Demux2/3 -> [ObjDxRL]
  Demux2/4 -> [ObjDxRR]
  Demux3/1 -> [ObjDyFR]
  Demux3/2 -> [ObjDyFL]
  Demux3/3 -> [ObjDyRL]
  Demux3/4 -> [ObjDyRR]
  [SubDxFR] -> Subsystem/1(SubDxFR)
  [SubDxFL] -> Subsystem/2(SubDxFL)
  [SubDxRL] -> Subsystem/3(SubDxRL)
  [SubDxRR] -> Subsystem/4(SubDxRR)
  [SubDxFR] -> Mux/1
  [SubDxFL] -> Mux/2
  Mux/1 -> Out1/1
  [SubDyFR] -> Subsystem1/1(SubDyFR)
  [SubDyFL] -> Subsystem1/2(SubDyFL)
  [SubDyRL] -> Subsystem1/3(SubDyRL)
  [SubDyRR] -> Subsystem1/4(SubDyRR)
  [ObjDxFR] -> Subsystem2/1(ObjDxFR)
  [ObjDxFL] -> Subsystem2/2(ObjDxFL)
  [ObjDxRL] -> Subsystem2/3(ObjDxRL)
  [ObjDxRR] -> Subsystem2/4(ObjDxRR)
  [ObjDyFR] -> Subsystem3/1(ObjDyFR)
  [ObjDyFL] -> Subsystem3/2(ObjDyFL)
  [ObjDyRL] -> Subsystem3/3(ObjDyRL)
  [ObjDyRR] -> Subsystem3/4(ObjDyRR)
  Subsystem/1(Out2) -> Mux/3
  Subsystem/2(Out1) -> Mux/4
  Subsystem1/1(Out2) -> Mux1/3
  Subsystem1/2(Out1) -> Mux1/4
  [SubDyFR] -> Mux1/1
  [SubDyFL] -> Mux1/2
  Subsystem2/1(Out2) -> Mux2/1
  Subsystem2/2(Out1) -> Mux2/2
  Subsystem3/1(Out2) -> Mux3/1
  Subsystem3/2(Out1) -> Mux3/2
  Mux1/1 -> Out2/1
  Mux2/1 -> Out3/1
  Mux3/1 -> Out4/1
  [ObjDxRL] -> Mux2/3
  [ObjDxRR] -> Mux2/4
  [ObjDyRL] -> Mux3/3
  [ObjDyRR] -> Mux3/4

== system TosAeb/AEB_Objsel/TA_Processor/AEB_ObjSelect/TargetInfoCheck/Data_precess/TA_EffectCalculate/Obj_FilterFlgCalculate/JA_ObjFilter/CollisionProbCalc/Subsystem/ObjPreReferPointCalc ==
  dx : Inport  OutDataTypeStr=single
  vxAbsObj : Inport  Port=2  OutDataTypeStr=single
  tPredTime : Inport  Port=3  OutDataTypeStr=single
  axAbsObj : Inport  Port=4  OutDataTypeStr=single
  vy : Inport  Port=5  OutDataTypeStr=single
  subjLatAccel : Inport  Port=6  OutDataTypeStr=single
  ay : Inport  Port=7  OutDataTypeStr=single
  dy : Inport  Port=8  OutDataTypeStr=single
  ObjectYawAngle : Inport  Port=9  OutDataTypeStr=single
  Add : Sum  Inputs=+++  OutDataTypeStr=single
  Add1 : Sum  Inputs=+++  OutDataTypeStr=single
  Add2 : Sum  OutDataTypeStr=single
  Add3 : Sum  Inputs=+-
  Add4 : Sum
  Constant : Constant  Value=180  OutDataTypeStr=single
  Constant1 : Constant  Value=P_TA_PrmForVehRearAxleDisttoFrnt  OutDataTypeStr=single
  Degrees to Radians : simulink_extras/Transformations/Degrees to Radians
  Gain : Gain  Gain=0.5  OutDataTypeStr=single
  Gain1 : Gain  Gain=0.5  OutDataTypeStr=single
  Gain2 : Gain  Gain=-1  OutDataTypeStr=single
  Math Function : Math  Operator=square  SignedPower=on  OutDataTypeStr=single  SaturateOnIntegerOverflow=on
  Math Function1 : Math  Operator=square  SignedPower=on  OutDataTypeStr=single  SaturateOnIntegerOverflow=on
  Product{,1..3} (4 alike) : Product  OutDataTypeStr=single
  dxPreRefPointObj : Outport
  dyPreRefPointObj : Outport  Port=2
  YawAnglePreObj : Outport  Port=3
  wires:
  vxAbsObj/1 -> Product/1
  tPredTime/1 -> Product/2, Math Function/1, Math Function1/1, Product2/2
  Product/1 -> Add/2
  Math Function/1 -> Product1/1
  axAbsObj/1 -> Product1/2
  Product1/1 -> Gain/1
  Gain/1 -> Add/3
  Add/1 -> dxPreRefPointObj/1
  vy/1 -> Product2/1
  Math Function1/1 -> Product3/1
  Product3/1 -> Gain1/1
  Product2/1 -> Add1/1
  Gain1/1 -> Add1/2
  dy/1 -> Add1/3
  Add1/1 -> dyPreRefPointObj/1
  ObjectYawAngle/1 -> Add3/2
  subjLatAccel/1 -> Add2/1
  ay/1 -> Add2/2
  Add2/1 -> Product3/2
  Constant/1 -> Add3/1
  Add3/1 -> Degrees to Radians/1
  Degrees to Radians/1 -> Gain2/1
  Gain2/1 -> YawAnglePreObj/1
  dx/1 -> Add4/1
  Constant1/1 -> Add4/2  "dxFrontBumperToRearAxis"
  Add4/1 -> Add/1

== system TosAeb/AEB_Objsel/TA_Processor/AEB_ObjSelect/TargetInfoCheck/Data_precess/TA_EffectCalculate/Obj_FilterFlgCalculate/JA_ObjFilter/CollisionProbCalc/Subsystem/PreCrossFlgCalcFlow/VectorCrossAlgorithm ==
  x1 : Inport  OutDataTypeStr=single
  y1 : Inport  Port=2  OutDataTypeStr=single
  x2 : Inport  Port=3  OutDataTypeStr=single
  y2 : Inport  Port=4  OutDataTypeStr=single
  m1 : Inport  Port=5  OutDataTypeStr=single
  n1 : Inport  Port=6  OutDataTypeStr=single
  m2 : Inport  Port=7  OutDataTypeStr=single
  n2 : Inport  Port=8  OutDataTypeStr=single
  f : TriggerPort  TriggerType=function-call  VariantControl=Variant
      settings: CopyFcn=Stateflow.SLINSF.SimulinkMan.removeBlockPreDeleteFcn  PreDeleteFcn=Stateflow.SLINSF.SimulinkMan.unsafePredeleteFcn
  Abs : Abs
  Bus Creator : BusCreator  Inputs=8
  Bus Selector : BusSelector  OutputSignals=x1,x2,n2,n1,y2,y1,m2,m1
  Compare To Constant : simulink/Logic and Bit Operations/Compare To Constant  relop=<  const=1e-6  OutDataTypeStr=boolean
  If : If  IfExpression=u1
  If Action Subsystem : Subsystem  TreatAsAtomicUnit=on
  If Action Subsystem1 : Subsystem  TreatAsAtomicUnit=on
  Merge : Merge
  VectorMulit : Subsystem
  preCrossFlg : Outport
  wires:
  x1/1 -> Bus Creator/1  "x1"
  y1/1 -> Bus Creator/2  "y1"
  x2/1 -> Bus Creator/3  "x2"
  y2/1 -> Bus Creator/4  "y2"
  m1/1 -> Bus Creator/5  "m1"
  n1/1 -> Bus Creator/6  "n1"
  m2/1 -> Bus Creator/7  "m2"
  n2/1 -> Bus Creator/8  "n2"
  Bus Creator/1 -> If Action Subsystem1/2(Coordinations), Bus Selector/1
  VectorMulit/1(vectorMulit) -> If Action Subsystem1/1(vectorMulit), Abs/1
  Abs/1 -> Compare To Constant/1
  Compare To Constant/1 -> If/1
  If/1 -> If Action Subsystem/Action
  If/2 -> If Action Subsystem1/Action
  If Action Subsystem/1(CrossFlg) -> Merge/1
  If Action Subsystem1/1(CrossFlg) -> Merge/2
  Merge/1 -> preCrossFlg/1
  Bus Selector/1 -> VectorMulit/1(x1)  "<x1>"
  Bus Selector/2 -> VectorMulit/2(x2)  "<x2>"
  Bus Selector/3 -> VectorMulit/3(n2)  "<n2>"
  Bus Selector/4 -> VectorMulit/4(n1)  "<n1>"
  Bus Selector/5 -> VectorMulit/5(y2)  "<y2>"
  Bus Selector/6 -> VectorMulit/6(y1)  "<y1>"
  Bus Selector/7 -> VectorMulit/7(m2)  "<m2>"
  Bus Selector/8 -> VectorMulit/8(m1)  "<m1>"
